simulink model "okayestcontroller"  (R2023b)
settings: Solver=FixedStepAuto  StartTime=0.0  StopTime=400  FixedStep=0.05  RelTol=1e-3
model workspace (MAT data in the model file):
  Logfile_Data_Object : robotics.ros.BagSelection (value not decoded)
  Logfile_Path = '/Users/marystirlingbrown/Documents/Autonomous_Vehicles_and_Traffic/Autonomous-Vehicles-ACC-Project/2023_09_28_16_19_25_2T3MWRFVXLW056972microstrain_record.bag'
  Logfile_Playback_Duration = 200
  Logfile_Playback_Offset = 100
libraries: robotlib x6
settings Model: Creator=sprinkle  LastModifiedBy=marystirlingbrown
settings SolverCC: AlgebraicLoopSolver=TrustRegion
settings OptimizationCC: EnhancedBackFolding=on  PassReuseOutputArgsAs=Individual arguments  ZeroExternalMemoryAtStartup=off  ZeroInternalMemoryAtStartup=off  MaxStackSize=64  OptimizeBlockOrder=speed  DifferentSizesBufferReuse=on  OptimizationCustomize=off  EfficientTunableParamExpr=on
settings DebuggingCC: ParameterTunabilityLossMsg=error  UnconnectedInputMsg=warning  UnconnectedOutputMsg=warning  UnconnectedLineMsg=warning
settings HardwareCC: ProdBitPerPointer=32  ProdBitPerSizeT=32  ProdBitPerPtrDiffT=32  ProdLargestAtomicFloat=None  ProdIntDivRoundTo=Undefined  ProdEndianess=Unspecified  ProdWordSize=32  ProdHWDeviceType=Generic->Unspecified (assume 32-bit Generic)
settings ModelReferenceCC: EnableRefExpFcnMdlSchedulingChecks=on
settings SFSimCC: SimTargetLang=C++
settings RTWCC: Description=Embedded Coder  SystemTargetFile=ert.tlc  HardwareBoard=Robot Operating System (ROS)  TLCOptions="-aInlineSetEventsForThisBaseRateFcn=TLC_FALSE -aSuppressMultiTaskScheduler=TLC_FALSE  -aRateBasedStepFcn=0"  GenCodeOnly=on  TemplateMakefile=ert_default_tmf  Toolchain=Catkin  TargetLang=C++
settings CodeAppCC: ForceParamTrailComments=on  IgnoreCustomStorageClasses=off  OperatorAnnotations=on  SimulinkDataObjDesc=on  SFDataObjDesc=on  InsertBlockDesc=on
settings ERTTargetCC: SupportContinuousTime=on  SupportVariableSizeSignals=on

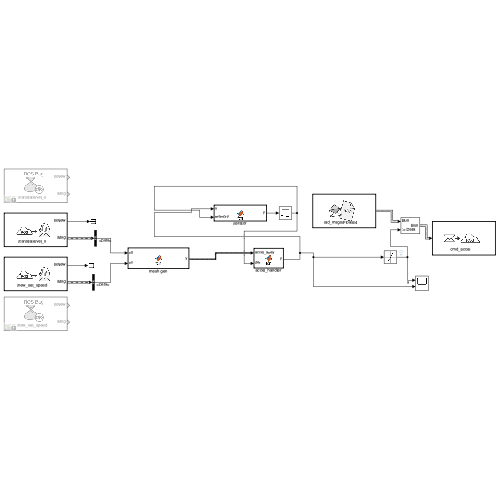
[thumbnail: Simulink's stored preview of the top-level diagram]
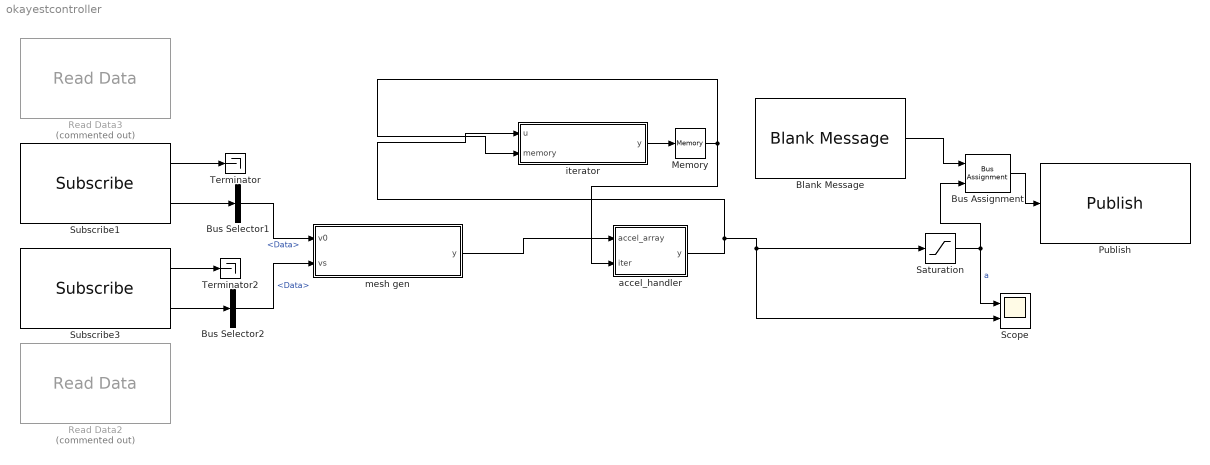
== system okayestcontroller ==
  Blank Message : robotlib/Blank Message  messageClass=Message  entityType=std_msgs/Float64  messageType=std_msgs/Float64  SampleTime=inf  legacyBusStruct=off
      settings: LibrarySourceBlock=roslib/ROS/Blank Message
  Bus Assignment : BusAssignment  AssignedSignals=Data
  Bus Selector1 : BusSelector  OutputSignals=Data
  Bus Selector2 : BusSelector  OutputSignals=Data
  Memory : Memory  InitialCondition=1
  Publish : robotlib/Publish  topicSource=Specify your own  topic=cmd_accel  messageType=std_msgs/Float64  legacyBusStruct=off  messageQueueLength=1
      settings: LibrarySourceBlock=roslib/ROS/Publish
  Read Data2 : robotlib/Read Data  Commented=on  topic=/new_set_speed  sampleTime=-1  logfileType=rosbag  modelName=okayestcontroller
  Read Data3 : robotlib/Read Data  Commented=on  topic=/car/state/vel_x  sampleTime=-1  logfileType=rosbag  modelName=okayestcontroller  msgType=std_msgs/Float64
  Saturation : Saturate  UpperLimit=1.5  LowerLimit=-3
  Scope : Scope  NumInputPorts=2
  Subscribe1 : robotlib/Subscribe  topicSource=Specify your own  topic=/car/state/vel_x  messageType=std_msgs/Float64  SampleTime=-1  legacyBusStruct=off  messageQueueLength=1
      settings: LibrarySourceBlock=roslib/ROS/Subscribe
  Subscribe3 : robotlib/Subscribe  topicSource=Specify your own  topic=/new_set_speed  messageType=std_msgs/Float64  SampleTime=-1  legacyBusStruct=off  messageQueueLength=1
      settings: LibrarySourceBlock=roslib/ROS/Subscribe
  Terminator : Terminator
  Terminator2 : Terminator
  accel_handler : MATLAB Function  TreatAsAtomicUnit=on
  iterator : MATLAB Function  TreatAsAtomicUnit=on
  mesh gen : MATLAB Function  TreatAsAtomicUnit=on
  wires:
  Subscribe1/1 -> Terminator/1
  Bus Assignment/1 -> Publish/1
  Bus Selector1/1 -> mesh gen/1(v0)  "<Data>"
  Bus Selector2/1 -> mesh gen/2(vs)  "<Data>"
  Saturation/1 -> Bus Assignment/2, Scope/1  "a"
  Subscribe3/1 -> Terminator2/1
  Blank Message/1 -> Bus Assignment/1
  Subscribe3/2 -> Bus Selector2/1
  Subscribe1/2 -> Bus Selector1/1
  accel_handler/1(y) -> Scope/2, Saturation/1, iterator/1(u)
  iterator/1(y) -> Memory/1
  Memory/1 -> iterator/2(memory), accel_handler/2(iter)
  mesh gen/1(y) -> accel_handler/1(accel_array)

== matlab function okayestcontroller/accel_handler ==
  data: accel_array input; y output; iter input
function y = fcn(accel_array, iter)

y = accel_array(iter)

== matlab function okayestcontroller/iterator ==
  settings: updateMethod=DISCRETE  sampleTime=0.1
  data: u input; y output; memory input
function y = fcn(u, memory)

iter = memory;



if u ~= 0 && iter < 100
    iter = iter + 1;
end


y = iter;

== matlab function okayestcontroller/mesh gen ==
  settings: updateMethod=DISCRETE  sampleTime=0.1
  data: v0 input; y output; vs input
function y = fcn(v0, vs)

    time = 10;          % time gap
    midpoint = time / 2; % describes midpoint of time

    % mesh_data = zeros(1000);
    mesh_data = zeros(1, 1000);
    if vs > v0
        % accelerate
        accel_mid = (vs - v0) / 2;
        mesh_data = [accel_mid];
        y_val = accel_mid;
        % build first part of acceleration mesh
        for i = 1:(midpoint * 10)
            % scaling with time to ensure convergence
            y_val = y_val / ((time + 1) / time);
            mesh_data = [y_val, mesh_data];
        end
        % Reset variables, declare second half of mesh
        y_val = accel_mid;
        sec_mesh = [];
        set_tmp = vs - v0;
        % construct second half of acceleration mesh
        for i = 1:(midpoint * 10)
            % remove next smallest increment obtained from set speed
            set_tmp = set_tmp - mesh_data(i);
            sec_mesh = [set_tmp, sec_mesh];
            set_tmp = vs - v0;
        end
        % concatenate meshes to form one mesh with h = 0.1
        mesh_data = [mesh_data, sec_mesh];
        
        y = mesh_data;
    elseif vs < v0
        % decelerate
        decel_mid = (v0 - vs) / 2;
        mesh_data = decel_mid;
        y_val = decel_mid;
        % build second half of mesh
        for i = 1:(midpoint * 10)
            % scale with time
            y_val = y_val / ((time + 1) / time);
            mesh_data = [mesh_data, y_val];
        end
        % Reset variables, init first half of mesh
        y_val = decel_mid;
        sec_mesh = [];
        set_tmp = v0 - vs;
        mesh_data = fliplr(mesh_data); % reversed to obtain smallest values first
        % similar in construction to first
        for i = 1:(midpoint * 10)
            set_tmp = set_tmp - mesh_data(i);
            sec_mesh = [sec_mesh, set_tmp];
            set_tmp = v0-vs;
        end
        % Reversed such that it decays from midpoint again
        mesh_data = fliplr(mesh_data);
        % Concatenate meshes
        mesh_data = [sec_mesh, mesh_data];
        y = mesh_data;
    else
        y = mesh_data;
    end

    my_spline = spline(0:0.1:10, mesh_data);
    derivative_array = [];
    a = 0.0;
    for idx = 1:length(mesh_data)  % Use an index variable instead of "for v = mesh_data"
        derivative_array = [derivative_array, (ppval(my_spline, a+0.1) - ppval(my_spline, a))/0.1];
        a = a + 0.1;
    end
    accel_array = derivative_array;
    % return accel_array
    y = accel_array;
end
